simulink model "TosAeb"  (R2021a)
settings: Solver=FixedStepDiscrete  StartTime=0.0  StopTime=inf  FixedStep=0.01  RelTol=1e-3
undefined symbols (not defined in the file or in its folder): ACCModSts, AccSts1, AEB_ObjectMovingOrientation, AEB_ObjectMovingState, CN_BTCActive, CN_CurrentGearR, CN_CurrentGearR_DHT, CN_PTCActive, CN_VDCActive, CN_VSC_EspOff, CN_VSC_FCW_AEB_Error, CN_VSC_FCW_AEB_NoDispay, CN_VSC_FCW_AEB_PerfmDgdtn, CN_VSC_FCW_AEB_TempUnAvlbl, CN_VSC_VehStandstill, CNaccelAvoidMax, CNaccelAvoidMin, CNbrakePedalPress, CNcomfortStrLeftAccelMax, CNcomfortStrLeftAccelMin, CNcomfortStrRightAccelMax, CNcomfortStrRightAccelMin, CNDrvDoorOpen, CNDrvModeEco, CNDrvModeNorm, CNDrvModeOther, CNDrvModeSport, CNDrvSBRreminder, CNNull, CNobjInvalidID, CNPassDoorOpen, CNPassSBRreminder, CNPEBSAccelNegInf, CNPEBSAccelNull, CNPEBSTimeInf, CNPEBSTimeNull, CNSTOPMAX, CNSTOPNULL, CNsubjWidth, CNSwtOFF, CNSwtON, CNTTCMAX, CNTTCNULL, CNVehConfigAEBCycNone, CNVehConfigAEBNone, CNVehConfigAEBPedNone, CNVehConfigFCWNone, CNVehConfigJANone, CollisionSeverity, FALSE, FCWSnvty, JACycObjCalcInfoDefaultParams, JAPedObjCalcInfoDefaultParams, JAVehObjCalcInfoDefaultParams, latCycObjCalcInfoDefaultParams, latPedObjCalcInfoDefaultParams, longObjCalcInfoDefaultParams, ModSts, movingStatus, ObjectType ... (+1112)
libraries: simulink x3031, AEBSFunctionLib x50, Ta_Library x33, AEBSStateMachineLib x23, Mylibrary x12, simulink_extras x7, ObjSelect_lib x3, dspstat3 x2
(part 48 of 102)

== system TosAeb/FcwAebJa_Step_sys/ALG_AEBSystem/ALG_AEBController/AEB_LatObjInfoCalcApp/LatCycObjCalc/LatCycCollisonAssessInfoCalc/LatCycCollisonAssessInfoCalc/DistBrkCondCalc ==
  note: 修改信息：
      | 替换distBrake计算模块
      | 20200420
  subLongAccel : Inport
  subjVel : Inport  Port=2
  CycDistBrakeFullCalc : Subsystem
  CycDistBrakePartCalc : Subsystem
  distBraking4FullBrakeCalc1 : Subsystem  Commented=on
  distBraking4PartBrakeCalc1 : Subsystem  Commented=on
  distSecurCalc : Subsystem
  distBraking4PartBrake : Outport
  distBraking4FullBrake : Outport  Port=2
  distSecure : Outport  Port=3
  wires:
  subLongAccel/1 -> CycDistBrakePartCalc/1(subjLongAccel), CycDistBrakeFullCalc/1(subjLongAccel)
  subjVel/1 -> CycDistBrakePartCalc/2(subjVel), distSecurCalc/1(subVel), CycDistBrakeFullCalc/2(subjVel)  "subjVel"
  CycDistBrakeFullCalc/1(distBrake) -> distBraking4FullBrake/1  "distBraking4FullBrake"
  distSecurCalc/1(distSecure) -> distSecure/1  "distSecure"
  CycDistBrakePartCalc/1(distBrake) -> distBraking4PartBrake/1  "distBraking4PartBrake"

== system TosAeb/FcwAebJa_Step_sys/ALG_AEBSystem/ALG_AEBController/AEB_LatObjInfoCalcApp/LatCycObjCalc/LatCycCollisonAssessInfoCalc/LatCycCollisonAssessInfoCalc/LatCollsionSeverityJudge ==
  ObjectID : Inport
  cycColliisonRiskProb : Inport  Port=2  OutDataTypeStr=single
  LatCollsionSeverityJudgeFlow : Stateflow chart  TreatAsAtomicUnit=on
  P_LatCycCA_Lvl1SeverityThresCurrentLvl0 : Constant  Value=P_AEB_LatCycCA_Lvl1SeverityThresCurrentLvl0
  P_LatCycCA_Lvl1SeverityThresCurrentLvl1 : Constant  Value=P_AEB_LatCycCA_Lvl1SeverityThresCurrentLvl1
  P_LatCycCA_Lvl1SeverityThresCurrentLvl2 : Constant  Value=P_AEB_LatCycCA_Lvl1SeverityThresCurrentLvl2
  P_LatCycCA_Lvl1SeverityThresCurrentLvl3 : Constant  Value=P_AEB_LatCycCA_Lvl1SeverityThresCurrentLvl3
  P_LatCycCA_Lvl2SeverityThresCurrentLvl0 : Constant  Value=P_AEB_LatCycCA_Lvl2SeverityThresCurrentLvl0
  P_LatCycCA_Lvl2SeverityThresCurrentLvl1 : Constant  Value=P_AEB_LatCycCA_Lvl2SeverityThresCurrentLvl1
  P_LatCycCA_Lvl2SeverityThresCurrentLvl2 : Constant  Value=P_AEB_LatCycCA_Lvl2SeverityThresCurrentLvl2
  P_LatCycCA_Lvl2SeverityThresCurrentLvl3 : Constant  Value=P_AEB_LatCycCA_Lvl2SeverityThresCurrentLvl3
  P_LatCycCA_Lvl3SeverityThresCurrentLvl0 : Constant  Value=P_AEB_LatCycCA_Lvl3SeverityThresCurrentLvl0
  P_LatCycCA_Lvl3SeverityThresCurrentLvl1 : Constant  Value=P_AEB_LatCycCA_Lvl3SeverityThresCurrentLvl1
  P_LatCycCA_Lvl3SeverityThresCurrentLvl2 : Constant  Value=P_AEB_LatCycCA_Lvl3SeverityThresCurrentLvl2
  P_LatCycCA_Lvl3SeverityThresCurrentLvl3 : Constant  Value=P_AEB_LatCycCA_Lvl3SeverityThresCurrentLvl3
  Unit Delay : UnitDelay  InitialCondition=CollisionSeverity.None  SampleTime=-1  HasFrameUpgradeWarning=on
      settings: AttributesFormatString=InitialCondition:%<InitialCondition>
  latCollsionSeverity : Outport
  wires:
  ObjectID/1 -> LatCollsionSeverityJudgeFlow/1(ObjectID)
  cycColliisonRiskProb/1 -> LatCollsionSeverityJudgeFlow/2(cycColliisonRiskProb)
  Unit Delay/1 -> LatCollsionSeverityJudgeFlow/15(latCollisionSeverityK1)
  LatCollsionSeverityJudgeFlow/1(latCollsionSeverity) -> latCollsionSeverity/1, Unit Delay/1  "latCollsionSeverity"
  P_LatCycCA_Lvl1SeverityThresCurrentLvl0/1 -> LatCollsionSeverityJudgeFlow/3(P_LatCycCA_Lvl1SeverityThresCurrentLvl0)
  P_LatCycCA_Lvl2SeverityThresCurrentLvl0/1 -> LatCollsionSeverityJudgeFlow/4(P_LatCycCA_Lvl2SeverityThresCurrentLvl0)
  P_LatCycCA_Lvl3SeverityThresCurrentLvl0/1 -> LatCollsionSeverityJudgeFlow/5(P_LatCycCA_Lvl3SeverityThresCurrentLvl0)
  P_LatCycCA_Lvl1SeverityThresCurrentLvl1/1 -> LatCollsionSeverityJudgeFlow/6(P_LatCycCA_Lvl1SeverityThresCurrentLvl1)
  P_LatCycCA_Lvl2SeverityThresCurrentLvl1/1 -> LatCollsionSeverityJudgeFlow/7(P_LatCycCA_Lvl2SeverityThresCurrentLvl1)
  P_LatCycCA_Lvl3SeverityThresCurrentLvl1/1 -> LatCollsionSeverityJudgeFlow/8(P_LatCycCA_Lvl3SeverityThresCurrentLvl1)
  P_LatCycCA_Lvl1SeverityThresCurrentLvl2/1 -> LatCollsionSeverityJudgeFlow/9(P_LatCycCA_Lvl1SeverityThresCurrentLvl2)
  P_LatCycCA_Lvl2SeverityThresCurrentLvl2/1 -> LatCollsionSeverityJudgeFlow/10(P_LatCycCA_Lvl2SeverityThresCurrentLvl2)
  P_LatCycCA_Lvl3SeverityThresCurrentLvl2/1 -> LatCollsionSeverityJudgeFlow/11(P_LatCycCA_Lvl3SeverityThresCurrentLvl2)
  P_LatCycCA_Lvl1SeverityThresCurrentLvl3/1 -> LatCollsionSeverityJudgeFlow/12(P_LatCycCA_Lvl1SeverityThresCurrentLvl3)
  P_LatCycCA_Lvl2SeverityThresCurrentLvl3/1 -> LatCollsionSeverityJudgeFlow/13(P_LatCycCA_Lvl2SeverityThresCurrentLvl3)
  P_LatCycCA_Lvl3SeverityThresCurrentLvl3/1 -> LatCollsionSeverityJudgeFlow/14(P_LatCycCA_Lvl3SeverityThresCurrentLvl3)

== system TosAeb/FcwAebJa_Step_sys/ALG_AEBSystem/ALG_AEBController/AEB_LatObjInfoCalcApp/LatCycObjCalc/LatCycObjCalc/TgtPreSelectCalc/ObjIsInvalidJudge ==
  ObjectID : Inport
  Compare To Constant : simulink/Logic and Bit Operations/Compare To Constant  relop===  const=CNobjInvalidID  OutDataTypeStr=boolean
  ObjIsInvalid : Outport
  wires:
  Compare To Constant/1 -> ObjIsInvalid/1
  ObjectID/1 -> Compare To Constant/1

== system TosAeb/FcwAebJa_Step_sys/ALG_AEBSystem/ALG_AEBController/AEB_LatObjInfoCalcApp/LatCycObjCalc/LatCycObjCalc/TgtPreSelectCalc/TgtPreSelect ==
  dx : Inport
  dy : Inport  Port=2
  ObjectExistProb : Inport  Port=3
  dyVariance : Inport  Port=4
  ObjectMovingOrientation : Inport  Port=5
  ObjectType : Inport  Port=6
  ObjectTypeCameraConfirmed : Inport  Port=7
  vx : Inport  Port=8
  subjVel : Inport  Port=9
  Action Port : ActionPort  ActionPortLabel=else
  Abs : Abs
  Abs1 : Abs
  Compare To Constant : simulink/Logic and Bit Operations/Compare To Constant  relop=<  const=P_AEB_LatCycCA_PreSelectDxThres  OutDataTypeStr=boolean
  Compare To Constant1 : simulink/Logic and Bit Operations/Compare To Constant  relop=<  const=P_AEB_LatCycCA_PreSelectDyThres  OutDataTypeStr=boolean
  Compare To Constant2 : simulink/Logic and Bit Operations/Compare To Constant  relop=>  const=P_AEB_LatCycCA_PreSelectObjectExistProbThres  OutDataTypeStr=boolean
  Compare To Constant3 : simulink/Logic and Bit Operations/Compare To Constant  relop=<  const=P_AEB_LatCycCA_PreSelectDyVarThres  OutDataTypeStr=boolean
  Compare To Constant4 : simulink/Logic and Bit Operations/Compare To Constant  relop=<  const=P_AEB_LatCycCA_PreSelectVxThres  OutDataTypeStr=boolean
  Compare To Constant5 : simulink/Logic and Bit Operations/Compare To Constant  relop===  const=AEB_ObjectMovingOrientation.Crossing  OutDataTypeStr=boolean
  Compare To Constant6 : simulink/Logic and Bit Operations/Compare To Constant  relop===  const=TRUE  OutDataTypeStr=boolean
  Compare To Constant8 : simulink/Logic and Bit Operations/Compare To Constant  relop===  const=ObjectTypeCopy.BICYCLE  OutDataTypeStr=boolean
  Constant : Constant  Value=P_Global_DxvFrontBumper_m
  Constant1 : Constant  Value=0  OutDataTypeStr=single
  Logical Operator3 : Logic  Inputs=8  AllPortsSameDT=off  OutDataTypeStr=boolean
  MinMax : MinMax  Function=max  Inputs=2
  "Object Cond " : Subsystem  Commented=on
  Subtract : Sum  Inputs=+-
  Subtract1 : Sum
  ObjPreSelectCond : Outport
  wires:
  dy/1 -> Abs/1
  Abs/1 -> Compare To Constant1/1
  ObjectExistProb/1 -> Compare To Constant2/1
  dyVariance/1 -> Compare To Constant3/1
  ObjectMovingOrientation/1 -> Compare To Constant5/1
  Logical Operator3/1 -> ObjPreSelectCond/1
  Compare To Constant/1 -> Logical Operator3/1
  Compare To Constant1/1 -> Logical Operator3/2
  Compare To Constant2/1 -> Logical Operator3/3
  Compare To Constant3/1 -> Logical Operator3/4
  Compare To Constant4/1 -> Logical Operator3/5
  Compare To Constant5/1 -> Logical Operator3/6
  Abs1/1 -> Compare To Constant4/1
  ObjectType/1 -> Compare To Constant8/1
  ObjectTypeCameraConfirmed/1 -> Compare To Constant6/1
  Compare To Constant8/1 -> Logical Operator3/7
  Compare To Constant6/1 -> Logical Operator3/8
  dx/1 -> Subtract/1
  Subtract/1 -> MinMax/1
  Constant/1 -> Subtract/2
  Constant1/1 -> MinMax/2
  MinMax/1 -> Compare To Constant/1
  vx/1 -> Subtract1/1
  subjVel/1 -> Subtract1/2
  Subtract1/1 -> Abs1/1

== system TosAeb/FcwAebJa_Step_sys/ALG_AEBSystem/ALG_AEBController/AEB_LatObjInfoCalcApp/LatCycObjCalc/LatCycObjCalc/TgtPreSelectCalc/TgtPreSelectDefault ==
  Action Port : ActionPort  ActionPortLabel=if(u1)
  Constant : Constant  Value=FALSE
  ObjPreSelectCond : Outport
  wires:
  Constant/1 -> ObjPreSelectCond/1

== system TosAeb/FcwAebJa_Step_sys/ALG_AEBSystem/ALG_AEBController/AEB_LatObjInfoCalcApp/LatCycObjCalc/LatCycObjCalc/latCycObjInfoCalc/ObjColiisionRiskProbCalc ==
  ObjInfoRaw : Inport
  ObjAbsInfo : Inport  Port=2
  subjInfo : Inport  Port=3
  Bus Selector : BusSelector  OutputSignals=ObjectTypeCameraConfirmed,ObjectLength,ObjectWidth,ObjectYawAngle,ObjectMovingOrientation,vx,vy,dy,dx
  Bus Selector1 : BusSelector  OutputSignals=subjVel,subjYawRate
  Bus Selector2 : BusSelector  OutputSignals=dxFrontBumper,vxAbsObj
  CollisionRiskProbCalc : Subsystem
  CollisionRiskProbCalcFlow : Stateflow chart  TreatAsAtomicUnit=on
  P_LatCycCA_CollisionRiskProbPredTimeMax : Constant  Value=P_AEB_LatCycCA_CollisionRiskProbPredTimeMax  OutDataTypeStr=single
  P_LatCycCA_CollisionRiskProbStepTime : Constant  Value=P_AEB_LatCycCA_CollisionRiskProbStepTime  OutDataTypeStr=single
  cycCollisionRiskProb : Outport
  dxCollisionRiskProbOut : Outport  Port=2
  dyCollisionRiskProbOut : Outport  Port=3
  preCollisonMaxTime : Outport  Port=4
  wires:
  CollisionRiskProbCalcFlow/3(dyCollisionRiskProbOut) -> dyCollisionRiskProbOut/1
  CollisionRiskProbCalcFlow/2(dxCollisionRiskProbOut) -> dxCollisionRiskProbOut/1
  CollisionRiskProbCalc/2(dyCollisionRiskProb) -> CollisionRiskProbCalcFlow/4(dyCollisionRiskProb)
  CollisionRiskProbCalc/3(dxCollisionRiskProb) -> CollisionRiskProbCalcFlow/5(dxCollisionRiskProb)
  CollisionRiskProbCalcFlow/1(collisionRiskProbMax) -> cycCollisionRiskProb/1  "cycCollisionRiskProb"
  Bus Selector2/1 -> CollisionRiskProbCalc/13(dxFrontBumper)  "<dxFrontBumper>"
  ObjAbsInfo/1 -> Bus Selector2/1
  Bus Selector1/2 -> CollisionRiskProbCalc/12(subjYawRate)  "<subjYawRate>"
  Bus Selector1/1 -> CollisionRiskProbCalc/11(subjVel)  "<subjVel>"
  subjInfo/1 -> Bus Selector1/1
  Bus Selector/9 -> CollisionRiskProbCalc/10(dx)  "<dx>"
  Bus Selector/8 -> CollisionRiskProbCalc/9(dy)  "<dy>"
  Bus Selector/7 -> CollisionRiskProbCalc/8(vy)  "<vy>"
  Bus Selector/6 -> CollisionRiskProbCalc/7(vx)  "<vx>"
  Bus Selector/4 -> CollisionRiskProbCalc/5(ObjectYawAngle)  "<ObjectYawAngle>"
  Bus Selector/3 -> CollisionRiskProbCalc/4(ObjectWidth)  "<ObjectWidth>"
  Bus Selector/1 -> CollisionRiskProbCalc/2(ObjectTypeCameraConfirmed)  "<ObjectTypeCameraConfirmed>"
  Bus Selector/2 -> CollisionRiskProbCalc/3(ObjectLength)  "<ObjectLength>"
  ObjInfoRaw/1 -> Bus Selector/1
  CollisionRiskProbCalcFlow/4(preCollisonMaxTime) -> preCollisonMaxTime/1
  P_LatCycCA_CollisionRiskProbStepTime/1 -> CollisionRiskProbCalcFlow/1(stepTime)
  P_LatCycCA_CollisionRiskProbPredTimeMax/1 -> CollisionRiskProbCalcFlow/2(tMaxPredTime)
  [CollisionRiskProbCalcFunc] -> CollisionRiskProbCalc/Trigger
  CollisionRiskProbCalcFlow/6(CollisionRiskProbCalcFunc()) -> [CollisionRiskProbCalcFunc]
  CollisionRiskProbCalcFlow/5(tPredictTimeOut) -> CollisionRiskProbCalc/1(tPredTime)
  CollisionRiskProbCalc/1(collisionRiskProb) -> CollisionRiskProbCalcFlow/3(collisionRiskProb)
  Bus Selector2/2 -> CollisionRiskProbCalc/14(vxAbsObj)  "<vxAbsObj>"
  Bus Selector/5 -> CollisionRiskProbCalc/6(ObjectMovingOrientation)  "<ObjectMovingOrientation>"

== system TosAeb/FcwAebJa_Step_sys/ALG_AEBSystem/ALG_AEBController/AEB_LatObjInfoCalcApp/LatCycObjCalc/LatCycObjCalc/latCycObjInfoCalc/ObjCrossFlgCal ==
  subjInfo : Inport
  ObjInfoRaw : Inport  Port=2
  ObjAbsInfo : Inport  Port=3
  Bus Selector : BusSelector  OutputSignals=subjVel,subjLongAccel,subjLatAccel,subjYawRate
  Bus Selector1 : BusSelector  OutputSignals=ObjectTypeCameraConfirmed,ObjectLength,ObjectWidth,ObjectYawAngle,ObjectMovingOrientation,dx,vy,ay,dy
  Bus Selector2 : BusSelector  OutputSignals=vxAbsObj,axAbsObj
  CollisionProbCalcFlow : Stateflow chart  TreatAsAtomicUnit=on
  CycPreCollisionCalcFunc : Subsystem
  P_LatCycCA_PreCollisionPredTimeMax : Constant  Value=P_AEB_LatCycCA_PreCollisionPredTimeMax  OutDataTypeStr=single
  P_LatCycCA_PreCollisionStepTime : Constant  Value=P_AEB_LatCycCA_PreCollisionStepTime  OutDataTypeStr=single
  cycPreCollisionFlg : Outport
  TTC : Outport  Port=2
  wires:
  Bus Selector1/9 -> CycPreCollisionCalcFunc/14(dy)  "<dy>"
  Bus Selector1/8 -> CycPreCollisionCalcFunc/13(ay)  "<ay>"
  Bus Selector1/4 -> CycPreCollisionCalcFunc/9(ObjectYawAngle)  "<ObjectYawAngle>"
  Bus Selector1/7 -> CycPreCollisionCalcFunc/12(vy)  "<vy>"
  Bus Selector1/2 -> CycPreCollisionCalcFunc/7(ObjectLength)  "<ObjectLength>"
  Bus Selector1/6 -> CycPreCollisionCalcFunc/11(dx)  "<dx>"
  Bus Selector1/3 -> CycPreCollisionCalcFunc/8(ObjectWidth)  "<ObjectWidth>"
  Bus Selector2/1 -> CycPreCollisionCalcFunc/15(vxAbsObj)  "<vxAbsObj>"
  Bus Selector2/2 -> CycPreCollisionCalcFunc/16(axAbsObj)  "<axAbsObj>"
  ObjAbsInfo/1 -> Bus Selector2/1
  ObjInfoRaw/1 -> Bus Selector1/1
  Bus Selector/4 -> CycPreCollisionCalcFunc/5(subjYawRate)  "<subjYawRate>"
  Bus Selector/3 -> CycPreCollisionCalcFunc/4(subjLatAccel)  "<subjLatAccel>"
  Bus Selector/2 -> CycPreCollisionCalcFunc/3(subjLongAccel)  "<subjLongAccel>"
  Bus Selector/1 -> CycPreCollisionCalcFunc/2(subjVel)  "<subjVel>"
  subjInfo/1 -> Bus Selector/1
  [CrossFlg] -> CycPreCollisionCalcFunc/Trigger
  CycPreCollisionCalcFunc/1(pedPreCollisionFlg) -> CollisionProbCalcFlow/3(preCollisionProb), cycPreCollisionFlg/1
  CollisionProbCalcFlow/3(CollisionProbFunc()) -> [CrossFlg]
  CollisionProbCalcFlow/1(tPredictTimeOut) -> CycPreCollisionCalcFunc/1(tPredTime)
  Bus Selector1/5 -> CycPreCollisionCalcFunc/10(ObjectMovingOrientation)  "<ObjectMovingOrientation>"
  P_LatCycCA_PreCollisionStepTime/1 -> CollisionProbCalcFlow/1(stepTime)
  P_LatCycCA_PreCollisionPredTimeMax/1 -> CollisionProbCalcFlow/2(tMaxPredTime)
  Bus Selector1/1 -> CycPreCollisionCalcFunc/6(ObjectTypeCameraConfirmed)  "<ObjectTypeCameraConfirmed>"
  CollisionProbCalcFlow/2(TTC) -> TTC/1

== system TosAeb/FcwAebJa_Step_sys/ALG_AEBSystem/ALG_AEBController/AEB_LatObjInfoCalcApp/LatCycObjCalc/LatCycObjCalc/latCycObjInfoCalc/SensorTgtInfo2AbsTgtInfoTrans ==
  dx : Inport
  vx : Inport  Port=2
  ax : Inport  Port=3
  vy : Inport  Port=4
  ObjectID : Inport  Port=5
  ObjectTypeCameraConfirmed : Inport  Port=6
  dy : Inport  Port=7
  FR_ObjectID : Inport  Port=8
  FR_Dx : Inport  Port=9
  FR_Dy : Inport  Port=10
  FR_Vy : Inport  Port=11
  FC_ObjectID : Inport  Port=12
  FC_Dx : Inport  Port=13
  FC_Dy : Inport  Port=14
  FC_Vy : Inport  Port=15
  subjVel : Inport  Port=16
  subjLongAccel : Inport  Port=17
  subjYawRate : Inport  Port=18
  Add : Sum
  Constant : Constant  Value=P_Global_DxvFrontBumper_m
  Constant1 : Constant  Value=0  OutDataTypeStr=single
  Constant2 : Constant  Value=P_AEB_LatCycObjAbs_SensorFR_VyThres
  Constant3 : Constant  Value=P_AEB_LatCycObjAbs_DyThres4deltaVy
  MinMax : MinMax  Function=max  Inputs=2
  ObjFusQualityCal : Stateflow chart  TreatAsAtomicUnit=on
  Product : Product
  Subtract : Sum  Inputs=+-
  Subtract1 : Sum
  Subtract2 : Sum
  dxFrontBumper : Outport
  vxAbsObj : Outport  Port=2
  axAbsObj : Outport  Port=3
  vyCorr : Outport  Port=4
  ObjFusQuality : Outport  Port=5
  wires:
  dx/1 -> Product/1, Subtract/1
  Subtract/1 -> MinMax/1
  vx/1 -> Subtract1/2
  subjVel/1 -> ObjFusQualityCal/14(subjVel), Subtract1/1
  Subtract1/1 -> vxAbsObj/1  "vxAbsObj"
  ax/1 -> Subtract2/1
  subjLongAccel/1 -> Subtract2/2
  Subtract2/1 -> axAbsObj/1  "axAbsObj"
  Constant/1 -> Subtract/2
  Constant1/1 -> MinMax/2
  MinMax/1 -> dxFrontBumper/1  "dxFrontBumper"
  Add/1 -> vyCorr/1  "vyCorr"
  subjYawRate/1 -> Product/2
  Product/1 -> Add/1
  vy/1 -> Add/2
  ObjFusQualityCal/1(ObjFusQuality) -> ObjFusQuality/1
  ObjectID/1 -> ObjFusQualityCal/1(ObjectID)
  ObjectTypeCameraConfirmed/1 -> ObjFusQualityCal/2(ObjectTypeCameraConfirmed)
  FC_ObjectID/1 -> ObjFusQualityCal/8(FC_ObjectID)
  FR_ObjectID/1 -> ObjFusQualityCal/4(FR_ObjectID)
  Constant2/1 -> ObjFusQualityCal/13(P_AEB_LatCycObjAbs_SensorFR_VyThres)
  FC_Vy/1 -> ObjFusQualityCal/11(FC_Vy)
  FR_Vy/1 -> ObjFusQualityCal/7(FR_Vy)
  FR_Dx/1 -> ObjFusQualityCal/5(FR_Dx)
  FC_Dx/1 -> ObjFusQualityCal/9(FC_Dx)
  FC_Dy/1 -> ObjFusQualityCal/10(FC_Dy)
  FR_Dy/1 -> ObjFusQualityCal/6(FR_Dy)
  Constant3/1 -> ObjFusQualityCal/12(P_AEB_LatCycObjAbs_DyThres4deltaVy)
  dy/1 -> ObjFusQualityCal/3(dy)

== system TosAeb/FcwAebJa_Step_sys/ALG_AEBSystem/ALG_AEBController/AEB_LatObjInfoCalcApp/LatPedObjCalc/LatPedCollisonAssessInfo/LatPedCollisonAssessInfo/LatPedCollisionSeverityCalc ==
  ObjectID : Inport
  pedColliisonRisk : Inport  Port=2  OutDataTypeStr=single
  dxFrontBumper : Inport  Port=3  OutDataTypeStr=single
  vx : Inport  Port=4
  subjVel : Inport  Port=5
  FcwHMISetting : Inport  Port=6  OutDataTypeStr=Enum: FCWSnvty
  distBraking : Inport  Port=7  OutDataTypeStr=single
  distSecure : Inport  Port=8
  Bus Creator : BusCreator  Inputs=6
  Bus Selector3 : BusSelector  OutputSignals=distSecure,distBraking,dxFrontBumper,vx
  Bus Selector4 : BusSelector  OutputSignals=FcwHMISetting,subjVel,dxFrontBumper
  Bus Selector5 : BusSelector  OutputSignals=dxFrontBumper,distSecure,distBraking,subjVel
  LatCollsionSeverityJudgeFlow : Stateflow chart  TreatAsAtomicUnit=on
  P_LatPedCA_Lvl1SeverityThresCurrentLevel0 : Constant  Value=P_AEB_LatPedCA_Lvl1SeverityThresCurrentLevel0
  P_LatPedCA_Lvl1SeverityThresCurrentLvl1 : Constant  Value=P_AEB_LatPedCA_Lvl1SeverityThresCurrentLvl1
  P_LatPedCA_Lvl1SeverityThresCurrentLvl2 : Constant  Value=P_AEB_LatPedCA_Lvl1SeverityThresCurrentLvl2
  P_LatPedCA_Lvl1SeverityThresCurrentLvl3 : Constant  Value=P_AEB_LatPedCA_Lvl1SeverityThresCurrentLvl3
  P_LatPedCA_Lvl2SeverityThresCurrentLvl0 : Constant  Value=P_AEB_LatPedCA_Lvl2SeverityThresCurrentLvl0
  P_LatPedCA_Lvl2SeverityThresCurrentLvl1 : Constant  Value=P_AEB_LatPedCA_Lvl2SeverityThresCurrentLvl1
  P_LatPedCA_Lvl2SeverityThresCurrentLvl2 : Constant  Value=P_AEB_LatPedCA_Lvl2SeverityThresCurrentLvl2
  P_LatPedCA_Lvl2SeverityThresCurrentLvl3 : Constant  Value=P_AEB_LatPedCA_Lvl2SeverityThresCurrentLvl3
  P_LatPedCA_Lvl3SeverityThresCurrentLvl0 : Constant  Value=P_AEB_LatPedCA_Lvl3SeverityThresCurrentLvl0
  P_LatPedCA_Lvl3SeverityThresCurrentLvl1 : Constant  Value=P_AEB_LatPedCA_Lvl3SeverityThresCurrentLvl1
  P_LatPedCA_Lvl3SeverityThresCurrentLvl2 : Constant  Value=P_AEB_LatPedCA_Lvl3SeverityThresCurrentLvl2
  P_LatPedCA_Lvl3SeverityThresCurrentLvl3 : Constant  Value=P_AEB_LatPedCA_Lvl3SeverityThresCurrentLvl3
  Unit Delay : UnitDelay  InitialCondition=CollisionSeverity.None  SampleTime=-1  HasFrameUpgradeWarning=on
      settings: AttributesFormatString=InitialCondition:%<InitialCondition>
  longDistCond4CollisionSeverityHighCalc : Subsystem
  longDistCond4CollisionSeverityLowCalc : Subsystem
  longDistCond4CollisionSeverityMediumCalc : Subsystem
  latCollsionSeverity : Outport
  wires:
  LatCollsionSeverityJudgeFlow/1(latCollsionSeverity) -> latCollsionSeverity/1, Unit Delay/1
  Unit Delay/1 -> LatCollsionSeverityJudgeFlow/18(latCollsionSeverityK1)
  ObjectID/1 -> LatCollsionSeverityJudgeFlow/1(TargetID)
  pedColliisonRisk/1 -> LatCollsionSeverityJudgeFlow/2(pedColliisonRisk)
  Bus Selector5/2 -> longDistCond4CollisionSeverityLowCalc/2(distSecure)  "<distSecure>"
  distBraking/1 -> Bus Creator/5  "distBraking"
  Bus Selector5/4 -> longDistCond4CollisionSeverityLowCalc/4(subjVel)  "<subjVel>"
  Bus Selector3/2 -> longDistCond4CollisionSeverityHighCalc/2(distBraking)  "<distBraking>"
  Bus Selector5/1 -> longDistCond4CollisionSeverityLowCalc/1(dxFrontBumper)  "<dxFrontBumper>"
  Bus Selector3/3 -> longDistCond4CollisionSeverityHighCalc/3(dxFrontBumper)  "<dxFrontBumper>"
  Bus Selector3/1 -> longDistCond4CollisionSeverityHighCalc/1(distSecure)  "<distSecure>"
  Bus Selector4/3 -> longDistCond4CollisionSeverityMediumCalc/3(dxFrontBumper)  "<dxFrontBumper>"
  Bus Selector5/3 -> longDistCond4CollisionSeverityLowCalc/3(distBraking)  "<distBraking>"
  Bus Selector4/2 -> longDistCond4CollisionSeverityMediumCalc/2(subjVel)  "<subjVel>"
  FcwHMISetting/1 -> Bus Creator/4  "FcwHMISetting"
  Bus Selector4/1 -> longDistCond4CollisionSeverityMediumCalc/1(FcwHMISetting)  "<FcwHMISetting>"
  Bus Creator/1 -> Bus Selector5/1, Bus Selector3/1, Bus Selector4/1
  dxFrontBumper/1 -> Bus Creator/1  "dxFrontBumper"
  distSecure/1 -> Bus Creator/6  "distSecure"
  subjVel/1 -> Bus Creator/3  "subjVel"
  Bus Selector3/4 -> longDistCond4CollisionSeverityHighCalc/4(vx)  "<vx>"
  longDistCond4CollisionSeverityLowCalc/1(longDistCond4CollisionSeverityLow) -> LatCollsionSeverityJudgeFlow/3(longDistCond4CollisionSeverityLow)
  vx/1 -> Bus Creator/2  "vx"
  P_LatPedCA_Lvl1SeverityThresCurrentLevel0/1 -> LatCollsionSeverityJudgeFlow/6(P_LatPedCA_Lvl1SeverityThresCurrentLvl0)
  P_LatPedCA_Lvl2SeverityThresCurrentLvl0/1 -> LatCollsionSeverityJudgeFlow/7(P_LatPedCA_Lvl2SeverityThresCurrentLvl0)
  P_LatPedCA_Lvl3SeverityThresCurrentLvl0/1 -> LatCollsionSeverityJudgeFlow/8(P_LatPedCA_Lvl3SeverityThresCurrentLvl0)
  P_LatPedCA_Lvl1SeverityThresCurrentLvl1/1 -> LatCollsionSeverityJudgeFlow/9(P_LatPedCA_Lvl1SeverityThresCurrentLvl1)
  P_LatPedCA_Lvl2SeverityThresCurrentLvl1/1 -> LatCollsionSeverityJudgeFlow/10(P_LatPedCA_Lvl2SeverityThresCurrentLvl1)
  P_LatPedCA_Lvl3SeverityThresCurrentLvl1/1 -> LatCollsionSeverityJudgeFlow/11(P_LatPedCA_Lvl3SeverityThresCurrentLvl1)
  P_LatPedCA_Lvl3SeverityThresCurrentLvl2/1 -> LatCollsionSeverityJudgeFlow/14(P_LatPedCA_Lvl3SeverityThresCurrentLvl2)
  P_LatPedCA_Lvl1SeverityThresCurrentLvl2/1 -> LatCollsionSeverityJudgeFlow/12(P_LatPedCA_Lvl1SeverityThresCurrentLvl2)
  P_LatPedCA_Lvl2SeverityThresCurrentLvl2/1 -> LatCollsionSeverityJudgeFlow/13(P_LatPedCA_Lvl2SeverityThresCurrentLvl2)
  P_LatPedCA_Lvl1SeverityThresCurrentLvl3/1 -> LatCollsionSeverityJudgeFlow/15(P_LatPedCA_Lvl1SeverityThresCurrentLvl3)
  P_LatPedCA_Lvl2SeverityThresCurrentLvl3/1 -> LatCollsionSeverityJudgeFlow/16(P_LatPedCA_Lvl2SeverityThresCurrentLvl3)
  P_LatPedCA_Lvl3SeverityThresCurrentLvl3/1 -> LatCollsionSeverityJudgeFlow/17(P_LatPedCA_Lvl3SeverityThresCurrentLvl3)
  longDistCond4CollisionSeverityMediumCalc/1(longDistCond4CollisionSeverityMedium) -> LatCollsionSeverityJudgeFlow/4(longDistCond4CollisionSeverityMedium)
  longDistCond4CollisionSeverityHighCalc/1(longDistCond4CollisionSeverityHigh) -> LatCollsionSeverityJudgeFlow/5(longDistCond4CollisionSeverityHigh)

== system TosAeb/FcwAebJa_Step_sys/ALG_AEBSystem/ALG_AEBController/AEB_LatObjInfoCalcApp/LatPedObjCalc/LatPedCollisonAssessInfo/LatPedCollisonAssessInfo/LatPedLongDistCondCalc ==
  note: 修改信息：
      | 替换distBrake计算模块
      | 20200420
  subjVel : Inport
  subLongAccel : Inport  Port=2  OutDataTypeStr=single
  DistSecureCalc : Subsystem
  PedDistBrakeFullCalc : Subsystem
  distBraking : Outport
  distSecure : Outport  Port=2
  wires:
  subjVel/1 -> PedDistBrakeFullCalc/2(subjVel), DistSecureCalc/1(subjVel)  "subjVel"
  subLongAccel/1 -> PedDistBrakeFullCalc/1(subjLongAccel)  "subLongAccel"
  PedDistBrakeFullCalc/1(distBrake) -> distBraking/1  "distBraking"
  DistSecureCalc/1(distSecure) -> distSecure/1  "distSecure"

== system TosAeb/FcwAebJa_Step_sys/ALG_AEBSystem/ALG_AEBController/AEB_LatObjInfoCalcApp/LatPedObjCalc/LatPedObjCalc/ObjPreSelectCondCalc/ObjIsInvalidJudge ==
  ObjectID : Inport
  Compare To Constant : simulink/Logic and Bit Operations/Compare To Constant  relop===  const=CNobjInvalidID  OutDataTypeStr=boolean
  ObjIsInvalid : Outport
  wires:
  ObjectID/1 -> Compare To Constant/1
  Compare To Constant/1 -> ObjIsInvalid/1

== system TosAeb/FcwAebJa_Step_sys/ALG_AEBSystem/ALG_AEBController/AEB_LatObjInfoCalcApp/LatPedObjCalc/LatPedObjCalc/ObjPreSelectCondCalc/TgtPreSelect ==
  dx : Inport
  dy : Inport  Port=2
  ObjectExistProb : Inport  Port=3
  dyVariance : Inport  Port=4
  ObjectMovingOrientation : Inport  Port=5
  ObjectType : Inport  Port=6
  ObjectTypeCameraConfirmed : Inport  Port=7
  vx : Inport  Port=8
  subjVel : Inport  Port=9
  Action Port : ActionPort  ActionPortLabel=else
  Abs : Abs
  Abs1 : Abs
  Compare To Constant : simulink/Logic and Bit Operations/Compare To Constant  relop=<  const=P_AEB_LatPedCA_PreSelectDxThres  OutDataTypeStr=boolean
  Compare To Constant1 : simulink/Logic and Bit Operations/Compare To Constant  relop=<  const=P_AEB_LatPedCA_PreSelectDyThres  OutDataTypeStr=boolean
  Compare To Constant2 : simulink/Logic and Bit Operations/Compare To Constant  relop=>  const=P_AEB_LatPedCA_PreSelectObjectExistProbThres  OutDataTypeStr=boolean
  Compare To Constant3 : simulink/Logic and Bit Operations/Compare To Constant  relop=<  const=P_AEB_LatPedCA_PreSelectDyVarThres  OutDataTypeStr=boolean
  Compare To Constant4 : simulink/Logic and Bit Operations/Compare To Constant  relop=<  const=P_AEB_LatPedCA_PreSelectVxThres  OutDataTypeStr=boolean
  Compare To Constant5 : simulink/Logic and Bit Operations/Compare To Constant  relop===  const=AEB_ObjectMovingOrientation.Crossing  OutDataTypeStr=boolean
  Compare To Constant6 : simulink/Logic and Bit Operations/Compare To Constant  relop===  const=TRUE  OutDataTypeStr=boolean
  Compare To Constant7 : simulink/Logic and Bit Operations/Compare To Constant  relop===  const=ObjectTypeCopy.PEDESTRIAN  OutDataTypeStr=boolean
  Constant : Constant  Value=P_Global_DxvFrontBumper_m
  Constant1 : Constant  Value=0  OutDataTypeStr=single
  Logical Operator3 : Logic  Inputs=8  AllPortsSameDT=off  OutDataTypeStr=boolean
  MinMax : MinMax  Function=max  Inputs=2
  ObjectTypeCond : Subsystem  Commented=on
  Subtract : Sum  Inputs=+-
  Subtract1 : Sum
  ObjPreSelectCond : Outport
  wires:
  dy/1 -> Abs/1
  Abs/1 -> Compare To Constant1/1
  ObjectExistProb/1 -> Compare To Constant2/1
  dyVariance/1 -> Compare To Constant3/1
  ObjectMovingOrientation/1 -> Compare To Constant5/1
  Logical Operator3/1 -> ObjPreSelectCond/1
  Compare To Constant/1 -> Logical Operator3/1
  Compare To Constant1/1 -> Logical Operator3/2
  Compare To Constant2/1 -> Logical Operator3/3
  Compare To Constant3/1 -> Logical Operator3/4
  Compare To Constant4/1 -> Logical Operator3/5
  Compare To Constant5/1 -> Logical Operator3/6
  Abs1/1 -> Compare To Constant4/1
  ObjectType/1 -> Compare To Constant7/1
  ObjectTypeCameraConfirmed/1 -> Compare To Constant6/1
  Compare To Constant7/1 -> Logical Operator3/7
  Compare To Constant6/1 -> Logical Operator3/8
  Constant/1 -> Subtract/2
  Constant1/1 -> MinMax/2
  Subtract/1 -> MinMax/1
  dx/1 -> Subtract/1
  MinMax/1 -> Compare To Constant/1  "dxFrontBumper"
  Subtract1/1 -> Abs1/1  "vxAbsObj"
  vx/1 -> Subtract1/1
  subjVel/1 -> Subtract1/2

== system TosAeb/FcwAebJa_Step_sys/ALG_AEBSystem/ALG_AEBController/AEB_LatObjInfoCalcApp/LatPedObjCalc/LatPedObjCalc/ObjPreSelectCondCalc/TgtPreSelectDefault ==
  Action Port : ActionPort  ActionPortLabel=if(u1)
  Constant : Constant  Value=FALSE
  ObjPreSelectCond : Outport
  wires:
  Constant/1 -> ObjPreSelectCond/1

== system TosAeb/FcwAebJa_Step_sys/ALG_AEBSystem/ALG_AEBController/AEB_LatObjInfoCalcApp/LatPedObjCalc/LatPedObjCalc/latPedObjInfoCalc/ObjColiisionRiskProbCalc ==
  ObjInfoRaw : Inport
  ObjAbsInfo : Inport  Port=2
  subjInfo : Inport  Port=3
  Bus Selector : BusSelector  OutputSignals=ObjectTypeCameraConfirmed,ObjectLength,ObjectWidth,ObjectYawAngle,vx,vy,dy,dx,ObjectMovingOrientation
  Bus Selector1 : BusSelector  OutputSignals=subjVel,subjYawRate
  Bus Selector2 : BusSelector  OutputSignals=dxFrontBumper
  CollisionRiskProbCalc : Subsystem
  CollisionRiskProbCalcFlow : Stateflow chart  TreatAsAtomicUnit=on
  P_LatPedCA_CollisionRiskProbPredTimeMax : Constant  Value=P_AEB_LatPedCA_CollisionRiskProbPredTimeMax  OutDataTypeStr=single
  P_LatPedCA_CollisionRiskProbStepTime : Constant  Value=P_AEB_LatPedCA_CollisionRiskProbStepTime  OutDataTypeStr=single
  pedCollisionRiskProb : Outport
  preCollisonMaxTime : Outport  Port=2
  dxCollisionRiskProbOut : Outport  Port=3
  dyCollisionRiskProbOut : Outport  Port=4
  wires:
  CollisionRiskProbCalcFlow/4(preCollisonMaxTime) -> preCollisonMaxTime/1
  CollisionRiskProbCalc/1(collisionRiskProb) -> CollisionRiskProbCalcFlow/3(collisionRiskProb)
  CollisionRiskProbCalcFlow/5(tPredictTimeOut) -> CollisionRiskProbCalc/1(tPredTime)
  CollisionRiskProbCalcFlow/6(CollisionRiskProbCalcFunc()) -> [CollisionRiskProbCalcFunc]
  [CollisionRiskProbCalcFunc] -> CollisionRiskProbCalc/Trigger
  P_LatPedCA_CollisionRiskProbPredTimeMax/1 -> CollisionRiskProbCalcFlow/2(tMaxPredTime)
  P_LatPedCA_CollisionRiskProbStepTime/1 -> CollisionRiskProbCalcFlow/1(stepTime)
  ObjInfoRaw/1 -> Bus Selector/1
  Bus Selector/2 -> CollisionRiskProbCalc/3(ObjectLength)  "<ObjectLength>"
  Bus Selector/1 -> CollisionRiskProbCalc/2(ObjectTypeCameraConfirmed)  "<ObjectTypeCameraConfirmed>"
  Bus Selector/3 -> CollisionRiskProbCalc/4(ObjectWidth)  "<ObjectWidth>"
  Bus Selector/4 -> CollisionRiskProbCalc/5(ObjectYawAngle)  "<ObjectYawAngle>"
  Bus Selector/5 -> CollisionRiskProbCalc/6(vx)  "<vx>"
  Bus Selector/6 -> CollisionRiskProbCalc/7(vy)  "<vy>"
  Bus Selector/7 -> CollisionRiskProbCalc/8(dy)  "<dy>"
  Bus Selector/8 -> CollisionRiskProbCalc/9(dx)  "<dx>"
  subjInfo/1 -> Bus Selector1/1
  Bus Selector1/1 -> CollisionRiskProbCalc/11(subjVel)  "<subjVel>"
  Bus Selector1/2 -> CollisionRiskProbCalc/12(subjYawRate)  "<subjYawRate>"
  ObjAbsInfo/1 -> Bus Selector2/1
  Bus Selector2/1 -> CollisionRiskProbCalc/13(dxFrontBumper)  "<dxFrontBumper>"
  CollisionRiskProbCalcFlow/1(collisionRiskProbMax) -> pedCollisionRiskProb/1  "pedCollisionRiskProb"
  CollisionRiskProbCalc/3(dxCollisionRiskProb) -> CollisionRiskProbCalcFlow/5(dxCollisionRiskProb)
  CollisionRiskProbCalc/2(dyCollisionRiskProb) -> CollisionRiskProbCalcFlow/4(dyCollisionRiskProb)
  CollisionRiskProbCalcFlow/2(dxCollisionRiskProbOut) -> dxCollisionRiskProbOut/1
  CollisionRiskProbCalcFlow/3(dyCollisionRiskProbOut) -> dyCollisionRiskProbOut/1
  Bus Selector/9 -> CollisionRiskProbCalc/10(ObjectMovingOrientation)  "<ObjectMovingOrientation>"

== system TosAeb/FcwAebJa_Step_sys/ALG_AEBSystem/ALG_AEBController/AEB_LatObjInfoCalcApp/LatPedObjCalc/LatPedObjCalc/latPedObjInfoCalc/ObjCrossFlgCal ==
  subjInfo : Inport
  ObjInfoRaw : Inport  Port=2
  ObjAbsInfo : Inport  Port=3
  Bus Selector : BusSelector  OutputSignals=subjVel,subjLongAccel,subjLatAccel,subjYawRate
  Bus Selector1 : BusSelector  OutputSignals=ObjectYawAngle,ObjectMovingOrientation,ObjectTypeCameraConfirmed,ObjectLength,ObjectWidth,dx,vy,ay,dy
  Bus Selector2 : BusSelector  OutputSignals=vxAbsObj,axAbsObj
  CollisionProbCalcFlow : Stateflow chart  TreatAsAtomicUnit=on
  P_LatPedCA_PreCollisionPredTimeMax : Constant  Value=P_AEB_LatPedCA_PreCollisionPredTimeMax  OutDataTypeStr=single
  P_LatPedCA_PreCollisionStepTime : Constant  Value=P_AEB_LatPedCA_PreCollisionStepTime  OutDataTypeStr=single
  PedPreCollisionCalcFunc : Subsystem
  pedPreCollisionFlg : Outport
  TTC : Outport  Port=2
  wires:
  CollisionProbCalcFlow/1(tPredictTimeOut) -> PedPreCollisionCalcFunc/1(tPredTime)
  CollisionProbCalcFlow/3(CollisionProbFunc()) -> [CrossFlg]
  PedPreCollisionCalcFunc/1(pedPreCollisionFlg) -> pedPreCollisionFlg/1, CollisionProbCalcFlow/3(preCollisionProb)
  P_LatPedCA_PreCollisionPredTimeMax/1 -> CollisionProbCalcFlow/2(tMaxPredTime)
  P_LatPedCA_PreCollisionStepTime/1 -> CollisionProbCalcFlow/1(stepTime)
  [CrossFlg] -> PedPreCollisionCalcFunc/Trigger
  subjInfo/1 -> Bus Selector/1
  Bus Selector/1 -> PedPreCollisionCalcFunc/2(subjVel)  "<subjVel>"
  Bus Selector/2 -> PedPreCollisionCalcFunc/3(subjLongAccel)  "<subjLongAccel>"
  Bus Selector/3 -> PedPreCollisionCalcFunc/4(subjLatAccel)  "<subjLatAccel>"
  Bus Selector/4 -> PedPreCollisionCalcFunc/5(subjYawRate)  "<subjYawRate>"
  ObjInfoRaw/1 -> Bus Selector1/1
  ObjAbsInfo/1 -> Bus Selector2/1
  Bus Selector2/2 -> PedPreCollisionCalcFunc/16(axAbsObj)  "<axAbsObj>"
  Bus Selector2/1 -> PedPreCollisionCalcFunc/15(vxAbsObj)  "<vxAbsObj>"
  Bus Selector1/5 -> PedPreCollisionCalcFunc/10(ObjectWidth)  "<ObjectWidth>"
  Bus Selector1/6 -> PedPreCollisionCalcFunc/11(dx)  "<dx>"
  Bus Selector1/4 -> PedPreCollisionCalcFunc/9(ObjectLength)  "<ObjectLength>"
  Bus Selector1/7 -> PedPreCollisionCalcFunc/12(vy)  "<vy>"
  Bus Selector1/1 -> PedPreCollisionCalcFunc/6(ObjectYawAngle)  "<ObjectYawAngle>"
  Bus Selector1/8 -> PedPreCollisionCalcFunc/13(ay)  "<ay>"
  Bus Selector1/9 -> PedPreCollisionCalcFunc/14(dy)  "<dy>"
  Bus Selector1/2 -> PedPreCollisionCalcFunc/7(ObjectMovingOrientation)  "<ObjectMovingOrientation>"
  Bus Selector1/3 -> PedPreCollisionCalcFunc/8(ObjectTypeCameraConfirmed)  "<ObjectTypeCameraConfirmed>"
  CollisionProbCalcFlow/2(TTC) -> TTC/1

== system TosAeb/FcwAebJa_Step_sys/ALG_AEBSystem/ALG_AEBController/AEB_LatObjInfoCalcApp/LatPedObjCalc/LatPedObjCalc/latPedObjInfoCalc/SensorTgtInfo2AbsTgtInfoTrans ==
  dx : Inport
  vx : Inport  Port=2
  ax : Inport  Port=3
  vy : Inport  Port=4
  ObjectID : Inport  Port=5
  ObjectTypeCameraConfirmed : Inport  Port=6
  dy : Inport  Port=7
  FR_ObjectID : Inport  Port=8
  FR_Dx : Inport  Port=9
  FR_Dy : Inport  Port=10
  FR_Vy : Inport  Port=11
  FC_ObjectID : Inport  Port=12
  FC_Dx : Inport  Port=13
  FC_Dy : Inport  Port=14
  FC_Vy : Inport  Port=15
  subjVel : Inport  Port=16
  subjLongAccel : Inport  Port=17
  subjYawRate : Inport  Port=18
  Add : Sum
  Constant : Constant  Value=P_Global_DxvFrontBumper_m
  Constant1 : Constant  Value=0  OutDataTypeStr=single
  Constant2 : Constant  Value=P_AEB_LatPedObjAbs_SensorFR_VyThres
  Constant3 : Constant  Value=P_AEB_LatPedObjAbs_DyThres4deltaVy
  MinMax : MinMax  Function=max  Inputs=2
  ObjFusQualityCal : Stateflow chart  TreatAsAtomicUnit=on
  Product : Product
  Subtract : Sum  Inputs=+-
  Subtract1 : Sum
  Subtract2 : Sum
  dxFrontBumper : Outport
  vxAbsObj : Outport  Port=2
  axAbsObj : Outport  Port=3
  vyCorr : Outport  Port=4
  ObjFusQuality : Outport  Port=5
  wires:
  dx/1 -> Product/1, Subtract/1
  Subtract/1 -> MinMax/1
  vx/1 -> Subtract1/2
  subjVel/1 -> ObjFusQualityCal/14(subjVel), Subtract1/1
  Subtract1/1 -> vxAbsObj/1  "vxAbsObj"
  ax/1 -> Subtract2/1
  subjLongAccel/1 -> Subtract2/2
  Subtract2/1 -> axAbsObj/1  "axAbsObj"
  Constant/1 -> Subtract/2
  Constant1/1 -> MinMax/2
  MinMax/1 -> dxFrontBumper/1  "dxFrontBumper"
  Add/1 -> vyCorr/1  "vyCorr"
  subjYawRate/1 -> Product/2
  Product/1 -> Add/1
  vy/1 -> Add/2
  ObjFusQualityCal/1(ObjFusQuality) -> ObjFusQuality/1
  ObjectID/1 -> ObjFusQualityCal/1(ObjectID)
  ObjectTypeCameraConfirmed/1 -> ObjFusQualityCal/2(ObjectTypeCameraConfirmed)
  FC_ObjectID/1 -> ObjFusQualityCal/8(FC_ObjectID)
  FR_ObjectID/1 -> ObjFusQualityCal/4(FR_ObjectID)
  Constant2/1 -> ObjFusQualityCal/13(P_AEB_LatPedObjAbs_SensorFR_VyThres)
  FC_Vy/1 -> ObjFusQualityCal/11(FC_Vy)
  FR_Vy/1 -> ObjFusQualityCal/7(FR_Vy)
  FR_Dx/1 -> ObjFusQualityCal/5(FR_Dx)
  FC_Dx/1 -> ObjFusQualityCal/9(FC_Dx)
  FR_Dy/1 -> ObjFusQualityCal/6(FR_Dy)
  FC_Dy/1 -> ObjFusQualityCal/10(FC_Dy)
  Constant3/1 -> ObjFusQualityCal/12(P_AEB_LatPedObjAbs_DyThres4deltaVy)
  dy/1 -> ObjFusQualityCal/3(dy)

== system TosAeb/FcwAebJa_Step_sys/ALG_AEBSystem/ALG_AEBController/AEB_LatObjInfoCalcApp/LatPedObjCalc/Subsystem/ObjPreSelectCondCalc/ObjIsInvalidJudge ==
  ObjectID : Inport
  Compare To Constant : simulink/Logic and Bit Operations/Compare To Constant  relop===  const=CNobjInvalidID  OutDataTypeStr=boolean
  ObjIsInvalid : Outport
  wires:
  ObjectID/1 -> Compare To Constant/1
  Compare To Constant/1 -> ObjIsInvalid/1

== system TosAeb/FcwAebJa_Step_sys/ALG_AEBSystem/ALG_AEBController/AEB_LatObjInfoCalcApp/LatPedObjCalc/Subsystem/ObjPreSelectCondCalc/TgtPreSelect ==
  dx : Inport
  dy : Inport  Port=2
  ObjectExistProb : Inport  Port=3
  dyVariance : Inport  Port=4
  ObjectMovingOrientation : Inport  Port=5
  ObjectType : Inport  Port=6
  ObjectTypeCameraConfirmed : Inport  Port=7
  vx : Inport  Port=8
  subjVel : Inport  Port=9
  Action Port : ActionPort  ActionPortLabel=else
  Abs : Abs
  Abs1 : Abs
  Compare To Constant : simulink/Logic and Bit Operations/Compare To Constant  relop=<  const=P_AEB_LatPedCA_PreSelectDxThres  OutDataTypeStr=boolean
  Compare To Constant1 : simulink/Logic and Bit Operations/Compare To Constant  relop=<  const=P_AEB_LatPedCA_PreSelectDyThres  OutDataTypeStr=boolean
  Compare To Constant2 : simulink/Logic and Bit Operations/Compare To Constant  relop=>  const=P_AEB_LatPedCA_PreSelectObjectExistProbThres  OutDataTypeStr=boolean
  Compare To Constant3 : simulink/Logic and Bit Operations/Compare To Constant  relop=<  const=P_AEB_LatPedCA_PreSelectDyVarThres  OutDataTypeStr=boolean
  Compare To Constant4 : simulink/Logic and Bit Operations/Compare To Constant  relop=<  const=P_AEB_LatPedCA_PreSelectVxThres  OutDataTypeStr=boolean
  Compare To Constant5 : simulink/Logic and Bit Operations/Compare To Constant  relop===  const=AEB_ObjectMovingOrientation.Crossing  OutDataTypeStr=boolean
  Compare To Constant6 : simulink/Logic and Bit Operations/Compare To Constant  relop===  const=TRUE  OutDataTypeStr=boolean
  Compare To Constant7 : simulink/Logic and Bit Operations/Compare To Constant  relop===  const=ObjectTypeCopy.PEDESTRIAN  OutDataTypeStr=boolean
  Constant : Constant  Value=P_Global_DxvFrontBumper_m
  Constant1 : Constant  Value=0  OutDataTypeStr=single
  Logical Operator3 : Logic  Inputs=8  AllPortsSameDT=off  OutDataTypeStr=boolean
  MinMax : MinMax  Function=max  Inputs=2
  ObjectTypeCond : Subsystem  Commented=on
  Subtract : Sum  Inputs=+-
  Subtract1 : Sum
  ObjPreSelectCond : Outport
  wires:
  dy/1 -> Abs/1
  Abs/1 -> Compare To Constant1/1
  ObjectExistProb/1 -> Compare To Constant2/1
  dyVariance/1 -> Compare To Constant3/1
  ObjectMovingOrientation/1 -> Compare To Constant5/1
  Logical Operator3/1 -> ObjPreSelectCond/1
  Compare To Constant/1 -> Logical Operator3/1
  Compare To Constant1/1 -> Logical Operator3/2
  Compare To Constant2/1 -> Logical Operator3/3
  Compare To Constant3/1 -> Logical Operator3/4
  Compare To Constant4/1 -> Logical Operator3/5
  Compare To Constant5/1 -> Logical Operator3/6
  Abs1/1 -> Compare To Constant4/1
  ObjectType/1 -> Compare To Constant7/1
  ObjectTypeCameraConfirmed/1 -> Compare To Constant6/1
  Compare To Constant7/1 -> Logical Operator3/7
  Compare To Constant6/1 -> Logical Operator3/8
  Constant/1 -> Subtract/2
  Constant1/1 -> MinMax/2
  Subtract/1 -> MinMax/1
  dx/1 -> Subtract/1
  MinMax/1 -> Compare To Constant/1  "dxFrontBumper"
  Subtract1/1 -> Abs1/1  "vxAbsObj"
  vx/1 -> Subtract1/1
  subjVel/1 -> Subtract1/2

== system TosAeb/FcwAebJa_Step_sys/ALG_AEBSystem/ALG_AEBController/AEB_LatObjInfoCalcApp/LatPedObjCalc/Subsystem/ObjPreSelectCondCalc/TgtPreSelectDefault ==
  Action Port : ActionPort  ActionPortLabel=if(u1)
  Constant : Constant  Value=FALSE
  ObjPreSelectCond : Outport
  wires:
  Constant/1 -> ObjPreSelectCond/1

== system TosAeb/FcwAebJa_Step_sys/ALG_AEBSystem/ALG_AEBController/AEB_LatObjInfoCalcApp/LatPedObjCalc/Subsystem/latPedObjInfoCalc/ObjColiisionRiskProbCalc ==
  ObjInfoRaw : Inport
  ObjAbsInfo : Inport  Port=2
  subjInfo : Inport  Port=3
  Bus Selector : BusSelector  OutputSignals=ObjectTypeCameraConfirmed,ObjectLength,ObjectWidth,ObjectYawAngle,vx,vy,dy,dx,ObjectMovingOrientation
  Bus Selector1 : BusSelector  OutputSignals=subjVel,subjYawRate
  Bus Selector2 : BusSelector  OutputSignals=dxFrontBumper
  CollisionRiskProbCalc : Subsystem
  CollisionRiskProbCalcFlow : Stateflow chart  TreatAsAtomicUnit=on
  P_LatPedCA_CollisionRiskProbPredTimeMax : Constant  Value=P_AEB_LatPedCA_CollisionRiskProbPredTimeMax  OutDataTypeStr=single
  P_LatPedCA_CollisionRiskProbStepTime : Constant  Value=P_AEB_LatPedCA_CollisionRiskProbStepTime  OutDataTypeStr=single
  pedCollisionRiskProb : Outport
  preCollisonMaxTime : Outport  Port=2
  dxCollisionRiskProbOut : Outport  Port=3
  dyCollisionRiskProbOut : Outport  Port=4
  wires:
  CollisionRiskProbCalcFlow/4(preCollisonMaxTime) -> preCollisonMaxTime/1
  CollisionRiskProbCalc/1(collisionRiskProb) -> CollisionRiskProbCalcFlow/3(collisionRiskProb)
  CollisionRiskProbCalcFlow/5(tPredictTimeOut) -> CollisionRiskProbCalc/1(tPredTime)
  CollisionRiskProbCalcFlow/6(CollisionRiskProbCalcFunc()) -> [CollisionRiskProbCalcFunc]
  [CollisionRiskProbCalcFunc] -> CollisionRiskProbCalc/Trigger
  P_LatPedCA_CollisionRiskProbPredTimeMax/1 -> CollisionRiskProbCalcFlow/2(tMaxPredTime)
  P_LatPedCA_CollisionRiskProbStepTime/1 -> CollisionRiskProbCalcFlow/1(stepTime)
  ObjInfoRaw/1 -> Bus Selector/1
  Bus Selector/2 -> CollisionRiskProbCalc/3(ObjectLength)  "<ObjectLength>"
  Bus Selector/1 -> CollisionRiskProbCalc/2(ObjectTypeCameraConfirmed)  "<ObjectTypeCameraConfirmed>"
  Bus Selector/3 -> CollisionRiskProbCalc/4(ObjectWidth)  "<ObjectWidth>"
  Bus Selector/4 -> CollisionRiskProbCalc/5(ObjectYawAngle)  "<ObjectYawAngle>"
  Bus Selector/5 -> CollisionRiskProbCalc/6(vx)  "<vx>"
  Bus Selector/6 -> CollisionRiskProbCalc/7(vy)  "<vy>"
  Bus Selector/7 -> CollisionRiskProbCalc/8(dy)  "<dy>"
  Bus Selector/8 -> CollisionRiskProbCalc/9(dx)  "<dx>"
  subjInfo/1 -> Bus Selector1/1
  Bus Selector1/1 -> CollisionRiskProbCalc/11(subjVel)  "<subjVel>"
  Bus Selector1/2 -> CollisionRiskProbCalc/12(subjYawRate)  "<subjYawRate>"
  ObjAbsInfo/1 -> Bus Selector2/1
  Bus Selector2/1 -> CollisionRiskProbCalc/13(dxFrontBumper)  "<dxFrontBumper>"
  CollisionRiskProbCalcFlow/1(collisionRiskProbMax) -> pedCollisionRiskProb/1  "pedCollisionRiskProb"
  CollisionRiskProbCalc/3(dxCollisionRiskProb) -> CollisionRiskProbCalcFlow/5(dxCollisionRiskProb)
  CollisionRiskProbCalc/2(dyCollisionRiskProb) -> CollisionRiskProbCalcFlow/4(dyCollisionRiskProb)
  CollisionRiskProbCalcFlow/2(dxCollisionRiskProbOut) -> dxCollisionRiskProbOut/1
  CollisionRiskProbCalcFlow/3(dyCollisionRiskProbOut) -> dyCollisionRiskProbOut/1
  Bus Selector/9 -> CollisionRiskProbCalc/10(ObjectMovingOrientation)  "<ObjectMovingOrientation>"

== system TosAeb/FcwAebJa_Step_sys/ALG_AEBSystem/ALG_AEBController/AEB_LatObjInfoCalcApp/LatPedObjCalc/Subsystem/latPedObjInfoCalc/ObjCrossFlgCal ==
  subjInfo : Inport
  ObjInfoRaw : Inport  Port=2
  ObjAbsInfo : Inport  Port=3
  Bus Selector : BusSelector  OutputSignals=subjVel,subjLongAccel,subjLatAccel,subjYawRate
  Bus Selector1 : BusSelector  OutputSignals=ObjectYawAngle,ObjectMovingOrientation,ObjectTypeCameraConfirmed,ObjectLength,ObjectWidth,dx,vy,ay,dy
  Bus Selector2 : BusSelector  OutputSignals=vxAbsObj,axAbsObj
  CollisionProbCalcFlow : Stateflow chart  TreatAsAtomicUnit=on
  P_LatPedCA_PreCollisionPredTimeMax : Constant  Value=P_AEB_LatPedCA_PreCollisionPredTimeMax  OutDataTypeStr=single
  P_LatPedCA_PreCollisionStepTime : Constant  Value=P_AEB_LatPedCA_PreCollisionStepTime  OutDataTypeStr=single
  PedPreCollisionCalcFunc : Subsystem
  pedPreCollisionFlg : Outport
  TTC : Outport  Port=2
  wires:
  CollisionProbCalcFlow/1(tPredictTimeOut) -> PedPreCollisionCalcFunc/1(tPredTime)
  CollisionProbCalcFlow/3(CollisionProbFunc()) -> [CrossFlg]
  PedPreCollisionCalcFunc/1(pedPreCollisionFlg) -> pedPreCollisionFlg/1, CollisionProbCalcFlow/3(preCollisionProb)
  P_LatPedCA_PreCollisionPredTimeMax/1 -> CollisionProbCalcFlow/2(tMaxPredTime)
  P_LatPedCA_PreCollisionStepTime/1 -> CollisionProbCalcFlow/1(stepTime)
  [CrossFlg] -> PedPreCollisionCalcFunc/Trigger
  subjInfo/1 -> Bus Selector/1
  Bus Selector/1 -> PedPreCollisionCalcFunc/2(subjVel)  "<subjVel>"
  Bus Selector/2 -> PedPreCollisionCalcFunc/3(subjLongAccel)  "<subjLongAccel>"
  Bus Selector/3 -> PedPreCollisionCalcFunc/4(subjLatAccel)  "<subjLatAccel>"
  Bus Selector/4 -> PedPreCollisionCalcFunc/5(subjYawRate)  "<subjYawRate>"
  ObjInfoRaw/1 -> Bus Selector1/1
  ObjAbsInfo/1 -> Bus Selector2/1
  Bus Selector2/2 -> PedPreCollisionCalcFunc/16(axAbsObj)  "<axAbsObj>"
  Bus Selector2/1 -> PedPreCollisionCalcFunc/15(vxAbsObj)  "<vxAbsObj>"
  Bus Selector1/5 -> PedPreCollisionCalcFunc/10(ObjectWidth)  "<ObjectWidth>"
  Bus Selector1/6 -> PedPreCollisionCalcFunc/11(dx)  "<dx>"
  Bus Selector1/4 -> PedPreCollisionCalcFunc/9(ObjectLength)  "<ObjectLength>"
  Bus Selector1/7 -> PedPreCollisionCalcFunc/12(vy)  "<vy>"
  Bus Selector1/1 -> PedPreCollisionCalcFunc/6(ObjectYawAngle)  "<ObjectYawAngle>"
  Bus Selector1/8 -> PedPreCollisionCalcFunc/13(ay)  "<ay>"
  Bus Selector1/9 -> PedPreCollisionCalcFunc/14(dy)  "<dy>"
  Bus Selector1/2 -> PedPreCollisionCalcFunc/7(ObjectMovingOrientation)  "<ObjectMovingOrientation>"
  Bus Selector1/3 -> PedPreCollisionCalcFunc/8(ObjectTypeCameraConfirmed)  "<ObjectTypeCameraConfirmed>"
  CollisionProbCalcFlow/2(TTC) -> TTC/1

== system TosAeb/FcwAebJa_Step_sys/ALG_AEBSystem/ALG_AEBController/AEB_LatObjInfoCalcApp/LatPedObjCalc/Subsystem/latPedObjInfoCalc/SensorTgtInfo2AbsTgtInfoTrans ==
  dx : Inport
  vx : Inport  Port=2
  ax : Inport  Port=3
  vy : Inport  Port=4
  ObjectID : Inport  Port=5
  ObjectTypeCameraConfirmed : Inport  Port=6
  dy : Inport  Port=7
  FR_ObjectID : Inport  Port=8
  FR_Dx : Inport  Port=9
  FR_Dy : Inport  Port=10
  FR_Vy : Inport  Port=11
  FC_ObjectID : Inport  Port=12
  FC_Dx : Inport  Port=13
  FC_Dy : Inport  Port=14
  FC_Vy : Inport  Port=15
  subjVel : Inport  Port=16
  subjLongAccel : Inport  Port=17
  subjYawRate : Inport  Port=18
  Add : Sum
  Constant : Constant  Value=P_Global_DxvFrontBumper_m
  Constant1 : Constant  Value=0  OutDataTypeStr=single
  Constant2 : Constant  Value=P_AEB_LatPedObjAbs_SensorFR_VyThres
  Constant3 : Constant  Value=P_AEB_LatPedObjAbs_DyThres4deltaVy
  MinMax : MinMax  Function=max  Inputs=2
  ObjFusQualityCal : Stateflow chart  TreatAsAtomicUnit=on
  Product : Product
  Subtract : Sum  Inputs=+-
  Subtract1 : Sum
  Subtract2 : Sum
  dxFrontBumper : Outport
  vxAbsObj : Outport  Port=2
  axAbsObj : Outport  Port=3
  vyCorr : Outport  Port=4
  ObjFusQuality : Outport  Port=5
  wires:
  dx/1 -> Product/1, Subtract/1
  Subtract/1 -> MinMax/1
  vx/1 -> Subtract1/2
  subjVel/1 -> ObjFusQualityCal/14(subjVel), Subtract1/1
  Subtract1/1 -> vxAbsObj/1  "vxAbsObj"
  ax/1 -> Subtract2/1
  subjLongAccel/1 -> Subtract2/2
  Subtract2/1 -> axAbsObj/1  "axAbsObj"
  Constant/1 -> Subtract/2
  Constant1/1 -> MinMax/2
  MinMax/1 -> dxFrontBumper/1  "dxFrontBumper"
  Add/1 -> vyCorr/1  "vyCorr"
  subjYawRate/1 -> Product/2
  Product/1 -> Add/1
  vy/1 -> Add/2
  ObjFusQualityCal/1(ObjFusQuality) -> ObjFusQuality/1
  ObjectID/1 -> ObjFusQualityCal/1(ObjectID)
  ObjectTypeCameraConfirmed/1 -> ObjFusQualityCal/2(ObjectTypeCameraConfirmed)
  FC_ObjectID/1 -> ObjFusQualityCal/8(FC_ObjectID)
  FR_ObjectID/1 -> ObjFusQualityCal/4(FR_ObjectID)
  Constant2/1 -> ObjFusQualityCal/13(P_AEB_LatPedObjAbs_SensorFR_VyThres)
  FC_Vy/1 -> ObjFusQualityCal/11(FC_Vy)
  FR_Vy/1 -> ObjFusQualityCal/7(FR_Vy)
  FR_Dx/1 -> ObjFusQualityCal/5(FR_Dx)
  FC_Dx/1 -> ObjFusQualityCal/9(FC_Dx)
  FR_Dy/1 -> ObjFusQualityCal/6(FR_Dy)
  FC_Dy/1 -> ObjFusQualityCal/10(FC_Dy)
  Constant3/1 -> ObjFusQualityCal/12(P_AEB_LatPedObjAbs_DyThres4deltaVy)
  dy/1 -> ObjFusQualityCal/3(dy)

== system TosAeb/FcwAebJa_Step_sys/ALG_AEBSystem/ALG_AEBController/AEB_LongTgtInfoCalc/LongObjCalcInfoCalc/AEBS_LongCollisionAssesApp/DriverReactionTimeCalc/ACCStateMonitor ==
  ACCState : Inport  OutDataTypeStr=Enum: ACCModSts
  ACCStateMonitor : Stateflow chart  TreatAsAtomicUnit=on
  Compare To Constant : simulink/Logic and Bit Operations/Compare To Constant  relop===  const=ACCModSts.ActiveControlMode  OutDataTypeStr=boolean
  P_LongCA_tACCProlongTimeThes : Constant  Value=P_AEB_LongCA_tACCProlongTimeThes
  SystemSampleTime : Constant  Value=Ts
  Unit Delay3 : UnitDelay  SampleTime=-1  HasFrameUpgradeWarning=on
  timesincelastACC : Outport
  ACCMonitor : Outport  Port=2
  wires:
  Unit Delay3/1 -> ACCStateMonitor/2(ACCstateK1)
  ACCState/1 -> Compare To Constant/1
  P_LongCA_tACCProlongTimeThes/1 -> ACCStateMonitor/3(PreactionTimeProlongationTimeACC)
  ACCStateMonitor/1(timesincelastACC) -> timesincelastACC/1
  ACCStateMonitor/2(ACCMonitor) -> ACCMonitor/1  "ACCMonitor"
  SystemSampleTime/1 -> ACCStateMonitor/4(Ts)
  Compare To Constant/1 -> Unit Delay3/1, ACCStateMonitor/1(ACCstate)
